simulink library "Renault_Library"  (Simulink 9.2)
settings: Solver=ode3  StartTime=0.0  StopTime=10.0  FixedStep=0.020  RelTol=1e-3  EnableMultiTasking=on
undefined symbols (not defined in the file or in its folder): ABC, AssystemProposal, b, chkval, CoeffA_C, CoeffB_C, CoeffMean_C, ConfTime_C, DiagDelay_C, DividePercent, FailureConfActive_C, FallingEdgeDuration_C, HighLevelThresholdVoltage_C, HighLevelVoltage_C, HighLevelVoltageTunable_C, InitValue_C, InputVoltRangeMax_C, InputVoltRangeMin_C, LowActiveContact_C, LowLevelThresholdVoltage_C, LowLevelVoltage_C, MaxOperatingVoltage_C, MinOperatingVoltage_C, mV2V_conversion, OUT1, OutOfRangeHigh_C, OutOfRangeLow_C, PinSupplySaturationMax, PinSupplySaturationMin, Res1Value_C, Res2Value_C, Res3Value_C, RisingEdgeDuration_C, RSA_CANSignalFailMonitoring, SampleTime_C, service14Table, service19Table, service22Table, service24Table, service28Table, service2ETable, service2FTable, service31Table, service87Table, SizeXStateDID2FTable, SizeXTable14, SizeXTable19, SizeXTable22, SizeXTable24, SizeXTable28, SizeXTable2E, SizeXTable2F, SizeXTable31, SizeXTable87, SizeYStateDID2FTable, SizeYTable14, SizeYTable19, SizeYTable22, SizeYTable24, SizeYTable28 ... (+22)
undefined symbols (not defined in the file): BatteryVoltage_ActivationThreshold_C, FrameStatus_AbsentValue_C, FrameStatus_ConfAbsentValue_C, MemThreshold_C, NAMEOFTHEMODEL_SAMPLE_TIME_CST, NbAbs1_C, NbConfAbs1_C, NbConfPres1_C, NbPres1_C, NOMDUSIGNAL_FaultConfTime_C, NOMDUSIGNAL_FaultRehabTime_C, NOMDUSIGNAL_switchvalue_C, NOMDUSIGNAL_unavailablevalue_C, PeriodFrame1_C, ResetTime_C, WakeUpTime_C
libraries: $bdroot x112, simulink x75
(part 26 of 32)

== system Renault_Library/Services ECU/Diag/RSA_DiagnosticManagement/DIAGNOSTIC_SANCTION_TOP/Service19/SubFunction_In_Active_Session_Service19/SubsystemDefaultSession_19 ==
  Active_Session : Inport
  Number_Subfunction : Inport  Port=2
  Constant{1..3,8,11,13,15,18..20,22,24,27..28,34,36,40..41,43,45,47,58,61} (23 alike) : Constant  Value=true  OutDataTypeStr=boolean  SampleTime=-1
  Constant10 : Constant  Value=ReporDTCSnapshotRecordByDTCNumberSession1  OutDataTypeStr=Inherit: Inherit via back propagation  SampleTime=-1
  Constant12 : Constant  Value=ReportNumberOfDTCByStatusMaskSession1  OutDataTypeStr=Inherit: Inherit via back propagation  SampleTime=-1
  Constant14 : Constant  Value=ReportDTCByStatusMaskSession1  OutDataTypeStr=Inherit: Inherit via back propagation  SampleTime=-1
  Constant16 : Constant  Value=ReporDTCSnapshotIdentificationSession1  OutDataTypeStr=Inherit: Inherit via back propagation  SampleTime=-1
  Constant17 : Constant  Value=ReportDTCStoredDataByRecordNumberSession1  OutDataTypeStr=Inherit: Inherit via back propagation  SampleTime=-1
  Constant21 : Constant  Value=ReportNumberOfDTCBySeverityMaskRecordSession1  OutDataTypeStr=Inherit: Inherit via back propagation  SampleTime=-1
  Constant23 : Constant  Value=ReportDTCBySeverityMaskRecordSession1  OutDataTypeStr=Inherit: Inherit via back propagation  SampleTime=-1
  Constant25 : Constant  Value=ReportSeverityInformationOfDTCSession1  OutDataTypeStr=Inherit: Inherit via back propagation  SampleTime=-1
  Constant26 : Constant  Value=ReportMostRecentConfimedDTCSession1  OutDataTypeStr=Inherit: Inherit via back propagation  SampleTime=-1
  Constant29 : Constant  Value=10  OutDataTypeStr=Inherit: Inherit via back propagation  SampleTime=-1
  Constant30 : Constant  Value=ReportFirstTestFailedDTCSession1  OutDataTypeStr=Inherit: Inherit via back propagation  SampleTime=-1
  Constant31 : Constant  Value=9  OutDataTypeStr=Inherit: Inherit via back propagation  SampleTime=-1
  Constant32 : Constant  Value=8  OutDataTypeStr=Inherit: Inherit via back propagation  SampleTime=-1
  Constant33 : Constant  Value=7  OutDataTypeStr=Inherit: Inherit via back propagation  SampleTime=-1
  Constant35 : Constant  Value=ReportFirstConfirmedDTCSession1  OutDataTypeStr=Inherit: Inherit via back propagation  SampleTime=-1
  Constant37 : Constant  Value=ReportMostRecentTestFailedDTCSession1  OutDataTypeStr=Inherit: Inherit via back propagation  SampleTime=-1
  Constant38 : Constant  Value=ReportMirrorMemoryDTCByStatusMaskSession1  OutDataTypeStr=Inherit: Inherit via back propagation  SampleTime=-1
  Constant4 : Constant  Value=4  OutDataTypeStr=Inherit: Inherit via back propagation  SampleTime=-1
  Constant42 : Constant  Value=ReportNumberOfMirrorMemoryDTCByStatusMaskSession1  OutDataTypeStr=Inherit: Inherit via back propagation  SampleTime=-1
  Constant44 : Constant  Value=ReportNumberOfEmissionsRelatedOBDDTCByStatusMaskSession1  OutDataTypeStr=Inherit: Inherit via back propagation  SampleTime=-1
  Constant46 : Constant  Value=ReportEmissionsRelatedOBDDTCByStatusMaskSession1  OutDataTypeStr=Inherit: Inherit via back propagation  SampleTime=-1
  Constant48 : Constant  Value=20  OutDataTypeStr=Inherit: Inherit via back propagation  SampleTime=-1
  Constant49 : Constant  Value=19  OutDataTypeStr=Inherit: Inherit via back propagation  SampleTime=-1
  Constant5 : Constant  Value=5  OutDataTypeStr=Inherit: Inherit via back propagation  SampleTime=-1
  Constant50 : Constant  Value=18  OutDataTypeStr=Inherit: Inherit via back propagation  SampleTime=-1
  Constant51 : Constant  Value=17  OutDataTypeStr=Inherit: Inherit via back propagation  SampleTime=-1
  Constant52 : Constant  Value=14  OutDataTypeStr=Inherit: Inherit via back propagation  SampleTime=-1
  Constant53 : Constant  Value=15  OutDataTypeStr=Inherit: Inherit via back propagation  SampleTime=-1
  Constant54 : Constant  Value=13  OutDataTypeStr=Inherit: Inherit via back propagation  SampleTime=-1
  Constant55 : Constant  Value=12  OutDataTypeStr=Inherit: Inherit via back propagation  SampleTime=-1
  Constant56 : Constant  Value=11  OutDataTypeStr=Inherit: Inherit via back propagation  SampleTime=-1
  Constant57 : Constant  Value=ReportMirrorMemoryDTCExtendedDataRecordByDTCNumberSession1  OutDataTypeStr=Inherit: Inherit via back propagation  SampleTime=-1
  Constant59 : Constant  Value=16  OutDataTypeStr=Inherit: Inherit via back propagation  SampleTime=-1
  Constant6 : Constant  Value=3  OutDataTypeStr=Inherit: Inherit via back propagation  SampleTime=-1
  Constant60 : Constant  Value=ReportDTCFaultDetectionCounterSession1  OutDataTypeStr=Inherit: Inherit via back propagation  SampleTime=-1
  Constant62 : Constant  Value=ReportDTCWithPermanetStatusSession1  OutDataTypeStr=Inherit: Inherit via back propagation  SampleTime=-1
  Constant64 : Constant  Value=ReportWWHOBDDTCByMaskRecordSession1  OutDataTypeStr=Inherit: Inherit via back propagation  SampleTime=-1
  Constant65 : Constant  Value=2  OutDataTypeStr=Inherit: Inherit via back propagation  SampleTime=-1
  Constant67 : Constant  Value=85  OutDataTypeStr=Inherit: Inherit via back propagation  SampleTime=-1
  Constant68 : Constant  Value=66  OutDataTypeStr=Inherit: Inherit via back propagation  SampleTime=-1
  Constant69 : Constant  Value=21  OutDataTypeStr=Inherit: Inherit via back propagation  SampleTime=-1
  Constant7 : Constant  OutDataTypeStr=Inherit: Inherit via back propagation  SampleTime=-1
  Constant70 : Constant  Value=ReportWWHOBDDTCWithPermanentStatusSession1  OutDataTypeStr=Inherit: Inherit via back propagation  SampleTime=-1
  Constant74 : Constant  Value=ReportDTCExtendedDataRecordByDTCNumberSession1  OutDataTypeStr=Inherit: Inherit via back propagation  SampleTime=-1
  Constant76 : Constant  Value=6  OutDataTypeStr=Inherit: Inherit via back propagation  SampleTime=-1
  Constant9 : Constant  Value=ReportSupportedDTCSession1  OutDataTypeStr=Inherit: Inherit via back propagation  SampleTime=-1
  Logical Operator{1..22,24} (23 alike) : Logic  OutDataTypeStr=boolean
  LogicalOperator{,1..3,5..22,28} (23 alike) : Logic  OutDataTypeStr=boolean
  LogicalOperator4 : Logic  Operator=OR  Inputs=23  OutDataTypeStr=boolean
  RelationalOperator{4..8,10..48,51..52} (46 alike) : RelationalOperator  Operator===  OutDataTypeStr=boolean  RndMeth=Nearest  InputSameDT=on
  Out1 : Outport
  wires:
  Constant7/1 -> RelationalOperator7/2
  Constant12/1 -> RelationalOperator10/1
  Constant1/1 -> RelationalOperator10/2
  RelationalOperator10/1 -> LogicalOperator/1
  Constant24/1 -> RelationalOperator11/2
  Constant14/1 -> RelationalOperator11/1
  RelationalOperator11/1 -> LogicalOperator1/1
  Constant40/1 -> RelationalOperator12/2
  Constant16/1 -> RelationalOperator12/1
  RelationalOperator12/1 -> LogicalOperator2/1
  Constant10/1 -> RelationalOperator14/1
  Constant41/1 -> RelationalOperator14/2
  RelationalOperator14/1 -> LogicalOperator3/1
  Constant17/1 -> RelationalOperator13/1
  Constant43/1 -> RelationalOperator13/2
  RelationalOperator13/1 -> LogicalOperator6/1
  Logical Operator2/1 -> LogicalOperator1/2
  Logical Operator1/1 -> LogicalOperator/2
  Active_Session/1 -> Logical Operator10/2, Logical Operator22/2, Logical Operator19/2, Logical Operator21/2, Logical Operator20/2, Logical Operator18/2, Logical Operator17/2, Logical Operator13/2, Logical Operator15/2, Logical Operator14/2, Logical Operator16/2, Logical Operator12/2, Logical Operator8/2, Logical Operator9/2, Logical Operator11/2, Logical Operator7/2, Logical Operator6/2, Logical Operator24/2, Logical Operator5/2, Logical Operator4/2, Logical Operator3/2, Logical Operator2/2, Logical Operator1/2
  Constant65/1 -> RelationalOperator45/2
  RelationalOperator45/1 -> Logical Operator2/1
  Constant4/1 -> RelationalOperator4/2
  Constant5/1 -> RelationalOperator5/2
  RelationalOperator5/1 -> Logical Operator4/1
  Logical Operator4/1 -> LogicalOperator6/2
  Logical Operator3/1 -> LogicalOperator3/2
  Constant6/1 -> RelationalOperator6/2
  Logical Operator5/1 -> LogicalOperator2/2
  RelationalOperator7/1 -> Logical Operator1/1
  RelationalOperator4/1 -> Logical Operator3/1
  RelationalOperator6/1 -> Logical Operator5/1
  [Number_Subfunction] -> RelationalOperator7/1
  [Number_Subfunction] -> RelationalOperator45/1
  [Number_Subfunction] -> RelationalOperator6/1
  [Number_Subfunction] -> RelationalOperator4/1
  [Number_Subfunction] -> RelationalOperator5/1
  Constant74/1 -> RelationalOperator51/1
  Constant45/1 -> RelationalOperator51/2
  RelationalOperator51/1 -> LogicalOperator28/1
  Constant76/1 -> RelationalOperator52/2
  RelationalOperator52/1 -> Logical Operator24/1
  Logical Operator24/1 -> LogicalOperator28/2
  [Number_Subfunction] -> RelationalOperator52/1
  Constant33/1 -> RelationalOperator25/2
  Constant21/1 -> RelationalOperator8/1
  Constant47/1 -> RelationalOperator8/2
  RelationalOperator8/1 -> LogicalOperator5/1
  Constant58/1 -> RelationalOperator15/2
  Constant23/1 -> RelationalOperator15/1
  RelationalOperator15/1 -> LogicalOperator7/1
  Constant61/1 -> RelationalOperator16/2
  Constant25/1 -> RelationalOperator16/1
  RelationalOperator16/1 -> LogicalOperator8/1
  Constant9/1 -> RelationalOperator18/1
  Constant2/1 -> RelationalOperator18/2
  RelationalOperator18/1 -> LogicalOperator10/1
  Logical Operator7/1 -> LogicalOperator7/2
  Logical Operator6/1 -> LogicalOperator5/2
  Constant32/1 -> RelationalOperator20/2
  RelationalOperator20/1 -> Logical Operator7/1
  Constant29/1 -> RelationalOperator19/2
  Logical Operator9/1 -> LogicalOperator10/2
  Constant31/1 -> RelationalOperator24/2
  Logical Operator11/1 -> LogicalOperator8/2
  RelationalOperator25/1 -> Logical Operator6/1
  RelationalOperator19/1 -> Logical Operator9/1
  RelationalOperator24/1 -> Logical Operator11/1
  [Number_Subfunction] -> RelationalOperator25/1
  [Number_Subfunction] -> RelationalOperator20/1
  [Number_Subfunction] -> RelationalOperator24/1
  [Number_Subfunction] -> RelationalOperator19/1
  Constant56/1 -> RelationalOperator40/2
  Constant30/1 -> RelationalOperator17/1
  Constant11/1 -> RelationalOperator17/2
  RelationalOperator17/1 -> LogicalOperator9/1
  Constant3/1 -> RelationalOperator21/2
  Constant35/1 -> RelationalOperator21/1
  RelationalOperator21/1 -> LogicalOperator11/1
  Constant13/1 -> RelationalOperator22/2
  Constant37/1 -> RelationalOperator22/1
  RelationalOperator22/1 -> LogicalOperator13/1
  Constant26/1 -> RelationalOperator26/1
  Constant8/1 -> RelationalOperator26/2
  RelationalOperator26/1 -> LogicalOperator15/1
  Constant38/1 -> RelationalOperator23/1
  Constant15/1 -> RelationalOperator23/2
  RelationalOperator23/1 -> LogicalOperator17/1
  Logical Operator12/1 -> LogicalOperator11/2
  Logical Operator8/1 -> LogicalOperator9/2
  Constant55/1 -> RelationalOperator35/2
  RelationalOperator35/1 -> Logical Operator12/1
  Constant52/1 -> RelationalOperator34/2
  Constant53/1 -> RelationalOperator36/2
  RelationalOperator36/1 -> Logical Operator15/1
  Logical Operator15/1 -> LogicalOperator17/2
  Logical Operator14/1 -> LogicalOperator15/2
  Constant54/1 -> RelationalOperator39/2
  Logical Operator16/1 -> LogicalOperator13/2
  RelationalOperator40/1 -> Logical Operator8/1
  RelationalOperator34/1 -> Logical Operator14/1
  RelationalOperator39/1 -> Logical Operator16/1
  [Number_Subfunction] -> RelationalOperator40/1
  [Number_Subfunction] -> RelationalOperator35/1
  [Number_Subfunction] -> RelationalOperator39/1
  [Number_Subfunction] -> RelationalOperator34/1
  [Number_Subfunction] -> RelationalOperator36/1
  Constant57/1 -> RelationalOperator37/1
  Constant18/1 -> RelationalOperator37/2
  RelationalOperator37/1 -> LogicalOperator14/1
  Constant59/1 -> RelationalOperator38/2
  RelationalOperator38/1 -> Logical Operator13/1
  Logical Operator13/1 -> LogicalOperator14/2
  [Number_Subfunction] -> RelationalOperator38/1
  Constant51/1 -> RelationalOperator33/2
  Constant42/1 -> RelationalOperator41/1
  Constant19/1 -> RelationalOperator41/2
  RelationalOperator41/1 -> LogicalOperator16/1
  Constant20/1 -> RelationalOperator27/2
  Constant44/1 -> RelationalOperator27/1
  RelationalOperator27/1 -> LogicalOperator18/1
  Constant22/1 -> RelationalOperator28/2
  Constant46/1 -> RelationalOperator28/1
  RelationalOperator28/1 -> LogicalOperator19/1
  Constant60/1 -> RelationalOperator29/1
  Constant27/1 -> RelationalOperator29/2
  RelationalOperator29/1 -> LogicalOperator12/1
  Logical Operator18/1 -> LogicalOperator18/2
  Logical Operator17/1 -> LogicalOperator16/2
  Constant50/1 -> RelationalOperator31/2
  RelationalOperator31/1 -> Logical Operator18/1
  Constant48/1 -> RelationalOperator30/2
  Logical Operator19/1 -> LogicalOperator12/2
  Constant49/1 -> RelationalOperator32/2
  Logical Operator10/1 -> LogicalOperator19/2
  RelationalOperator33/1 -> Logical Operator17/1
  RelationalOperator30/1 -> Logical Operator19/1
  RelationalOperator32/1 -> Logical Operator10/1
  [Number_Subfunction] -> RelationalOperator33/1
  [Number_Subfunction] -> RelationalOperator31/1
  [Number_Subfunction] -> RelationalOperator32/1
  [Number_Subfunction] -> RelationalOperator30/1
  Constant28/1 -> RelationalOperator42/2
  Constant62/1 -> RelationalOperator42/1
  RelationalOperator42/1 -> LogicalOperator21/1
  Constant34/1 -> RelationalOperator43/2
  Constant64/1 -> RelationalOperator43/1
  RelationalOperator43/1 -> LogicalOperator22/1
  Constant70/1 -> RelationalOperator44/1
  Constant36/1 -> RelationalOperator44/2
  RelationalOperator44/1 -> LogicalOperator20/1
  Logical Operator21/1 -> LogicalOperator21/2
  Constant69/1 -> RelationalOperator47/2
  RelationalOperator47/1 -> Logical Operator21/1
  Constant67/1 -> RelationalOperator46/2
  Logical Operator22/1 -> LogicalOperator20/2
  Constant68/1 -> RelationalOperator48/2
  Logical Operator20/1 -> LogicalOperator22/2
  RelationalOperator46/1 -> Logical Operator22/1
  RelationalOperator48/1 -> Logical Operator20/1
  [Number_Subfunction] -> RelationalOperator47/1
  [Number_Subfunction] -> RelationalOperator48/1
  [Number_Subfunction] -> RelationalOperator46/1
  LogicalOperator/1 -> LogicalOperator4/1
  LogicalOperator1/1 -> LogicalOperator4/2
  LogicalOperator2/1 -> LogicalOperator4/3
  LogicalOperator3/1 -> LogicalOperator4/4
  LogicalOperator6/1 -> LogicalOperator4/5
  LogicalOperator28/1 -> LogicalOperator4/6
  LogicalOperator5/1 -> LogicalOperator4/7
  LogicalOperator7/1 -> LogicalOperator4/8
  LogicalOperator8/1 -> LogicalOperator4/9
  LogicalOperator10/1 -> LogicalOperator4/10
  LogicalOperator9/1 -> LogicalOperator4/11
  LogicalOperator11/1 -> LogicalOperator4/12
  LogicalOperator13/1 -> LogicalOperator4/13
  LogicalOperator15/1 -> LogicalOperator4/14
  LogicalOperator17/1 -> LogicalOperator4/15
  LogicalOperator14/1 -> LogicalOperator4/16
  LogicalOperator16/1 -> LogicalOperator4/17
  LogicalOperator18/1 -> LogicalOperator4/18
  LogicalOperator19/1 -> LogicalOperator4/19
  LogicalOperator12/1 -> LogicalOperator4/20
  LogicalOperator21/1 -> LogicalOperator4/21
  LogicalOperator22/1 -> LogicalOperator4/22
  LogicalOperator20/1 -> LogicalOperator4/23
  LogicalOperator4/1 -> Out1/1
  Number_Subfunction/1 -> [Number_Subfunction]

== system Renault_Library/Services ECU/Diag/RSA_DiagnosticManagement/DIAGNOSTIC_SANCTION_TOP/Service19/SubFunction_In_Active_Session_Service19/SubsystemExtenderdSession_19 ==
  Active_Session : Inport
  Number_Subfunction : Inport  Port=2
  Constant{1..3,8,11,13,15,18..20,22,24,27..28,34,36,40..41,43,45,47,58,61} (23 alike) : Constant  Value=true  OutDataTypeStr=boolean  SampleTime=-1
  Constant144 : Constant  Value=ReportMostRecentTestFailedDTCSession3  OutDataTypeStr=Inherit: Inherit via back propagation  SampleTime=-1
  Constant145 : Constant  Value=ReportMirrorMemoryDTCByStatusMaskSession3  OutDataTypeStr=Inherit: Inherit via back propagation  SampleTime=-1
  Constant147 : Constant  Value=4  OutDataTypeStr=Inherit: Inherit via back propagation  SampleTime=-1
  Constant150 : Constant  Value=ReportNumberOfMirrorMemoryDTCByStatusMaskSession3  OutDataTypeStr=Inherit: Inherit via back propagation  SampleTime=-1
  Constant152 : Constant  Value=ReportNumberOfEmissionsRelatedOBDDTCByStatusMaskSession3  OutDataTypeStr=Inherit: Inherit via back propagation  SampleTime=-1
  Constant154 : Constant  Value=ReportEmissionsRelatedOBDDTCByStatusMaskSession3  OutDataTypeStr=Inherit: Inherit via back propagation  SampleTime=-1
  Constant156 : Constant  Value=20  OutDataTypeStr=Inherit: Inherit via back propagation  SampleTime=-1
  Constant157 : Constant  Value=19  OutDataTypeStr=Inherit: Inherit via back propagation  SampleTime=-1
  Constant158 : Constant  Value=5  OutDataTypeStr=Inherit: Inherit via back propagation  SampleTime=-1
  Constant159 : Constant  Value=18  OutDataTypeStr=Inherit: Inherit via back propagation  SampleTime=-1
  Constant160 : Constant  Value=17  OutDataTypeStr=Inherit: Inherit via back propagation  SampleTime=-1
  Constant161 : Constant  Value=14  OutDataTypeStr=Inherit: Inherit via back propagation  SampleTime=-1
  Constant162 : Constant  Value=15  OutDataTypeStr=Inherit: Inherit via back propagation  SampleTime=-1
  Constant163 : Constant  Value=13  OutDataTypeStr=Inherit: Inherit via back propagation  SampleTime=-1
  Constant164 : Constant  Value=12  OutDataTypeStr=Inherit: Inherit via back propagation  SampleTime=-1
  Constant165 : Constant  Value=11  OutDataTypeStr=Inherit: Inherit via back propagation  SampleTime=-1
  Constant166 : Constant  Value=ReportMirrorMemoryDTCExtendedDataRecordByDTCNumberSession3  OutDataTypeStr=Inherit: Inherit via back propagation  SampleTime=-1
  Constant168 : Constant  Value=16  OutDataTypeStr=Inherit: Inherit via back propagation  SampleTime=-1
  Constant169 : Constant  Value=3  OutDataTypeStr=Inherit: Inherit via back propagation  SampleTime=-1
  Constant170 : Constant  Value=ReportDTCFaultDetectionCounterSession3  OutDataTypeStr=Inherit: Inherit via back propagation  SampleTime=-1
  Constant172 : Constant  Value=ReportDTCWithPermanetStatusSession3  OutDataTypeStr=Inherit: Inherit via back propagation  SampleTime=-1
  Constant174 : Constant  Value=ReportWWHOBDDTCByMaskRecordSession3  OutDataTypeStr=Inherit: Inherit via back propagation  SampleTime=-1
  Constant175 : Constant  Value=2  OutDataTypeStr=Inherit: Inherit via back propagation  SampleTime=-1
  Constant177 : Constant  Value=85  OutDataTypeStr=Inherit: Inherit via back propagation  SampleTime=-1
  Constant178 : Constant  Value=66  OutDataTypeStr=Inherit: Inherit via back propagation  SampleTime=-1
  Constant179 : Constant  Value=21  OutDataTypeStr=Inherit: Inherit via back propagation  SampleTime=-1
  Constant180 : Constant  OutDataTypeStr=Inherit: Inherit via back propagation  SampleTime=-1
  Constant181 : Constant  Value=ReportWWHOBDDTCWithPermanentStatusSession3  OutDataTypeStr=Inherit: Inherit via back propagation  SampleTime=-1
  Constant182 : Constant  Value=ReportDTCExtendedDataRecordByDTCNumberSession3  OutDataTypeStr=Inherit: Inherit via back propagation  SampleTime=-1
  Constant184 : Constant  Value=6  OutDataTypeStr=Inherit: Inherit via back propagation  SampleTime=-1
  Constant185 : Constant  Value=ReportSupportedDTCSession3  OutDataTypeStr=Inherit: Inherit via back propagation  SampleTime=-1
  Constant186 : Constant  Value=ReporDTCSnapshotRecordByDTCNumberSession3  OutDataTypeStr=Inherit: Inherit via back propagation  SampleTime=-1
  Constant188 : Constant  Value=ReportNumberOfDTCByStatusMaskSession3  OutDataTypeStr=Inherit: Inherit via back propagation  SampleTime=-1
  Constant190 : Constant  Value=ReportDTCByStatusMaskSession3  OutDataTypeStr=Inherit: Inherit via back propagation  SampleTime=-1
  Constant192 : Constant  Value=ReporDTCSnapshotIdentificationSession3  OutDataTypeStr=Inherit: Inherit via back propagation  SampleTime=-1
  Constant193 : Constant  Value=ReportDTCStoredDataByRecordNumberSession3  OutDataTypeStr=Inherit: Inherit via back propagation  SampleTime=-1
  Constant197 : Constant  Value=ReportNumberOfDTCBySeverityMaskRecordSession3  OutDataTypeStr=Inherit: Inherit via back propagation  SampleTime=-1
  Constant199 : Constant  Value=ReportDTCBySeverityMaskRecordSession3  OutDataTypeStr=Inherit: Inherit via back propagation  SampleTime=-1
  Constant201 : Constant  Value=ReportSeverityInformationOfDTCSession3  OutDataTypeStr=Inherit: Inherit via back propagation  SampleTime=-1
  Constant202 : Constant  Value=ReportMostRecentConfimedDTCSession3  OutDataTypeStr=Inherit: Inherit via back propagation  SampleTime=-1
  Constant205 : Constant  Value=10  OutDataTypeStr=Inherit: Inherit via back propagation  SampleTime=-1
  Constant206 : Constant  Value=ReportFirstTestFailedDTCSession3  OutDataTypeStr=Inherit: Inherit via back propagation  SampleTime=-1
  Constant207 : Constant  Value=9  OutDataTypeStr=Inherit: Inherit via back propagation  SampleTime=-1
  Constant208 : Constant  Value=8  OutDataTypeStr=Inherit: Inherit via back propagation  SampleTime=-1
  Constant209 : Constant  Value=7  OutDataTypeStr=Inherit: Inherit via back propagation  SampleTime=-1
  Constant211 : Constant  Value=ReportFirstConfirmedDTCSession3  OutDataTypeStr=Inherit: Inherit via back propagation  SampleTime=-1
  Logical Operator{47..69} (23 alike) : Logic  OutDataTypeStr=boolean
  LogicalOperator{49..66,68..72} (23 alike) : Logic  OutDataTypeStr=boolean
  LogicalOperator67 : Logic  Operator=OR  Inputs=23  OutDataTypeStr=boolean
  RelationalOperator{97..142} (46 alike) : RelationalOperator  Operator===  OutDataTypeStr=boolean  RndMeth=Nearest  InputSameDT=on
  Out1 : Outport
  wires:
  Constant180/1 -> RelationalOperator141/2
  Constant188/1 -> RelationalOperator97/1
  Constant1/1 -> RelationalOperator97/2
  RelationalOperator97/1 -> LogicalOperator49/1
  Constant24/1 -> RelationalOperator98/2
  Constant190/1 -> RelationalOperator98/1
  RelationalOperator98/1 -> LogicalOperator50/1
  Constant40/1 -> RelationalOperator99/2
  Constant192/1 -> RelationalOperator99/1
  RelationalOperator99/1 -> LogicalOperator61/1
  Constant186/1 -> RelationalOperator101/1
  Constant41/1 -> RelationalOperator101/2
  RelationalOperator101/1 -> LogicalOperator66/1
  Constant193/1 -> RelationalOperator100/1
  Constant43/1 -> RelationalOperator100/2
  RelationalOperator100/1 -> LogicalOperator69/1
  Logical Operator58/1 -> LogicalOperator50/2
  Logical Operator47/1 -> LogicalOperator49/2
  Active_Session/1 -> Logical Operator48/2, Logical Operator61/2, Logical Operator57/2, Logical Operator60/2, Logical Operator59/2, Logical Operator56/2, Logical Operator55/2, Logical Operator51/2, Logical Operator53/2, Logical Operator52/2, Logical Operator54/2, Logical Operator50/2, Logical Operator68/2, Logical Operator69/2, Logical Operator49/2, Logical Operator67/2, Logical Operator66/2, Logical Operator62/2, Logical Operator65/2, Logical Operator64/2, Logical Operator63/2, Logical Operator58/2, Logical Operator47/2
  Constant175/1 -> RelationalOperator133/2
  RelationalOperator133/1 -> Logical Operator58/1
  Constant147/1 -> RelationalOperator127/2
  Constant158/1 -> RelationalOperator137/2
  RelationalOperator137/1 -> Logical Operator64/1
  Logical Operator64/1 -> LogicalOperator69/2
  Logical Operator63/1 -> LogicalOperator66/2
  Constant169/1 -> RelationalOperator140/2
  Logical Operator65/1 -> LogicalOperator61/2
  RelationalOperator141/1 -> Logical Operator47/1
  RelationalOperator127/1 -> Logical Operator63/1
  RelationalOperator140/1 -> Logical Operator65/1
  [Number_Subfunction] -> RelationalOperator141/1
  [Number_Subfunction] -> RelationalOperator133/1
  [Number_Subfunction] -> RelationalOperator140/1
  [Number_Subfunction] -> RelationalOperator127/1
  [Number_Subfunction] -> RelationalOperator137/1
  Constant182/1 -> RelationalOperator138/1
  ? -> RelationalOperator138/2
  RelationalOperator138/1 -> LogicalOperator65/1
  Constant184/1 -> RelationalOperator139/2
  RelationalOperator139/1 -> Logical Operator62/1
  Logical Operator62/1 -> LogicalOperator65/2
  [Number_Subfunction] -> RelationalOperator139/1
  Constant209/1 -> RelationalOperator112/2
  Constant197/1 -> RelationalOperator142/1
  Constant47/1 -> RelationalOperator142/2
  RelationalOperator142/1 -> LogicalOperator68/1
  Constant58/1 -> RelationalOperator102/2
  Constant199/1 -> RelationalOperator102/1
  RelationalOperator102/1 -> LogicalOperator70/1
  Constant61/1 -> RelationalOperator103/2
  Constant201/1 -> RelationalOperator103/1
  RelationalOperator103/1 -> LogicalOperator71/1
  Constant185/1 -> RelationalOperator105/1
  Constant2/1 -> RelationalOperator105/2
  RelationalOperator105/1 -> LogicalOperator51/1
  Logical Operator67/1 -> LogicalOperator70/2
  Logical Operator66/1 -> LogicalOperator68/2
  Constant208/1 -> RelationalOperator107/2
  RelationalOperator107/1 -> Logical Operator67/1
  Constant205/1 -> RelationalOperator106/2
  Logical Operator69/1 -> LogicalOperator51/2
  Constant207/1 -> RelationalOperator111/2
  Logical Operator49/1 -> LogicalOperator71/2
  RelationalOperator112/1 -> Logical Operator66/1
  RelationalOperator106/1 -> Logical Operator69/1
  RelationalOperator111/1 -> Logical Operator49/1
  [Number_Subfunction] -> RelationalOperator112/1
  [Number_Subfunction] -> RelationalOperator107/1
  [Number_Subfunction] -> RelationalOperator111/1
  [Number_Subfunction] -> RelationalOperator106/1
  Constant165/1 -> RelationalOperator128/2
  Constant206/1 -> RelationalOperator104/1
  Constant11/1 -> RelationalOperator104/2
  RelationalOperator104/1 -> LogicalOperator72/1
  Constant3/1 -> RelationalOperator108/2
  Constant211/1 -> RelationalOperator108/1
  RelationalOperator108/1 -> LogicalOperator52/1
  Constant13/1 -> RelationalOperator109/2
  Constant144/1 -> RelationalOperator109/1
  RelationalOperator109/1 -> LogicalOperator54/1
  Constant202/1 -> RelationalOperator113/1
  Constant8/1 -> RelationalOperator113/2
  RelationalOperator113/1 -> LogicalOperator56/1
  Constant145/1 -> RelationalOperator110/1
  Constant15/1 -> RelationalOperator110/2
  RelationalOperator110/1 -> LogicalOperator58/1
  Logical Operator50/1 -> LogicalOperator52/2
  Logical Operator68/1 -> LogicalOperator72/2
  Constant164/1 -> RelationalOperator122/2
  RelationalOperator122/1 -> Logical Operator50/1
  Constant161/1 -> RelationalOperator121/2
  Constant162/1 -> RelationalOperator123/2
  RelationalOperator123/1 -> Logical Operator53/1
  Logical Operator53/1 -> LogicalOperator58/2
  Logical Operator52/1 -> LogicalOperator56/2
  Constant163/1 -> RelationalOperator126/2
  Logical Operator54/1 -> LogicalOperator54/2
  RelationalOperator128/1 -> Logical Operator68/1
  RelationalOperator121/1 -> Logical Operator52/1
  RelationalOperator126/1 -> Logical Operator54/1
  [Number_Subfunction] -> RelationalOperator128/1
  [Number_Subfunction] -> RelationalOperator122/1
  [Number_Subfunction] -> RelationalOperator126/1
  [Number_Subfunction] -> RelationalOperator121/1
  [Number_Subfunction] -> RelationalOperator123/1
  Constant166/1 -> RelationalOperator124/1
  Constant18/1 -> RelationalOperator124/2
  RelationalOperator124/1 -> LogicalOperator55/1
  Constant168/1 -> RelationalOperator125/2
  RelationalOperator125/1 -> Logical Operator51/1
  Logical Operator51/1 -> LogicalOperator55/2
  [Number_Subfunction] -> RelationalOperator125/1
  Constant160/1 -> RelationalOperator120/2
  Constant150/1 -> RelationalOperator129/1
  Constant19/1 -> RelationalOperator129/2
  RelationalOperator129/1 -> LogicalOperator57/1
  Constant20/1 -> RelationalOperator114/2
  Constant152/1 -> RelationalOperator114/1
  RelationalOperator114/1 -> LogicalOperator59/1
  Constant22/1 -> RelationalOperator115/2
  Constant154/1 -> RelationalOperator115/1
  RelationalOperator115/1 -> LogicalOperator60/1
  Constant170/1 -> RelationalOperator116/1
  Constant27/1 -> RelationalOperator116/2
  RelationalOperator116/1 -> LogicalOperator53/1
  Logical Operator56/1 -> LogicalOperator59/2
  Logical Operator55/1 -> LogicalOperator57/2
  Constant159/1 -> RelationalOperator118/2
  RelationalOperator118/1 -> Logical Operator56/1
  Constant156/1 -> RelationalOperator117/2
  Logical Operator57/1 -> LogicalOperator53/2
  Constant157/1 -> RelationalOperator119/2
  Logical Operator48/1 -> LogicalOperator60/2
  RelationalOperator120/1 -> Logical Operator55/1
  RelationalOperator117/1 -> Logical Operator57/1
  RelationalOperator119/1 -> Logical Operator48/1
  [Number_Subfunction] -> RelationalOperator120/1
  [Number_Subfunction] -> RelationalOperator118/1
  [Number_Subfunction] -> RelationalOperator119/1
  [Number_Subfunction] -> RelationalOperator117/1
  Constant28/1 -> RelationalOperator130/2
  Constant172/1 -> RelationalOperator130/1
  RelationalOperator130/1 -> LogicalOperator63/1
  Constant34/1 -> RelationalOperator131/2
  Constant174/1 -> RelationalOperator131/1
  RelationalOperator131/1 -> LogicalOperator64/1
  Constant181/1 -> RelationalOperator132/1
  Constant36/1 -> RelationalOperator132/2
  RelationalOperator132/1 -> LogicalOperator62/1
  Logical Operator60/1 -> LogicalOperator63/2
  Constant179/1 -> RelationalOperator135/2
  RelationalOperator135/1 -> Logical Operator60/1
  Constant177/1 -> RelationalOperator134/2
  Logical Operator61/1 -> LogicalOperator62/2
  Constant178/1 -> RelationalOperator136/2
  Logical Operator59/1 -> LogicalOperator64/2
  RelationalOperator134/1 -> Logical Operator61/1
  RelationalOperator136/1 -> Logical Operator59/1
  [Number_Subfunction] -> RelationalOperator135/1
  [Number_Subfunction] -> RelationalOperator136/1
  [Number_Subfunction] -> RelationalOperator134/1
  LogicalOperator49/1 -> LogicalOperator67/1
  LogicalOperator50/1 -> LogicalOperator67/2
  LogicalOperator61/1 -> LogicalOperator67/3
  LogicalOperator66/1 -> LogicalOperator67/4
  LogicalOperator69/1 -> LogicalOperator67/5
  LogicalOperator65/1 -> LogicalOperator67/6
  LogicalOperator68/1 -> LogicalOperator67/7
  LogicalOperator70/1 -> LogicalOperator67/8
  LogicalOperator71/1 -> LogicalOperator67/9
  LogicalOperator51/1 -> LogicalOperator67/10
  LogicalOperator72/1 -> LogicalOperator67/11
  LogicalOperator52/1 -> LogicalOperator67/12
  LogicalOperator54/1 -> LogicalOperator67/13
  LogicalOperator56/1 -> LogicalOperator67/14
  LogicalOperator58/1 -> LogicalOperator67/15
  LogicalOperator55/1 -> LogicalOperator67/16
  LogicalOperator57/1 -> LogicalOperator67/17
  LogicalOperator59/1 -> LogicalOperator67/18
  LogicalOperator60/1 -> LogicalOperator67/19
  LogicalOperator53/1 -> LogicalOperator67/20
  LogicalOperator63/1 -> LogicalOperator67/21
  LogicalOperator64/1 -> LogicalOperator67/22
  LogicalOperator62/1 -> LogicalOperator67/23
  LogicalOperator67/1 -> Out1/1
  Number_Subfunction/1 -> [Number_Subfunction]

== system Renault_Library/Services ECU/Diag/RSA_DiagnosticManagement/DIAGNOSTIC_SANCTION_TOP/Service19/SubFunction_In_Active_Session_Service19/SubsystemProgrammingSession_19 ==
  Active_Session : Inport
  Number_Subfunction : Inport  Port=2
  Constant{1..3,8,11,13,15,18..20,22,24,27..28,34,36,40..41,43,45,47,58,61} (23 alike) : Constant  Value=true  OutDataTypeStr=boolean  SampleTime=-1
  Constant101 : Constant  Value=ReportMostRecentTestFailedDTCSession2  OutDataTypeStr=Inherit: Inherit via back propagation  SampleTime=-1
  Constant102 : Constant  Value=ReportMirrorMemoryDTCByStatusMaskSession2  OutDataTypeStr=Inherit: Inherit via back propagation  SampleTime=-1
  Constant104 : Constant  Value=4  OutDataTypeStr=Inherit: Inherit via back propagation  SampleTime=-1
  Constant107 : Constant  Value=ReportNumberOfMirrorMemoryDTCByStatusMaskSession2  OutDataTypeStr=Inherit: Inherit via back propagation  SampleTime=-1
  Constant109 : Constant  Value=ReportNumberOfEmissionsRelatedOBDDTCByStatusMaskSession2  OutDataTypeStr=Inherit: Inherit via back propagation  SampleTime=-1
  Constant111 : Constant  Value=ReportEmissionsRelatedOBDDTCByStatusMaskSession2  OutDataTypeStr=Inherit: Inherit via back propagation  SampleTime=-1
  Constant113 : Constant  Value=20  OutDataTypeStr=Inherit: Inherit via back propagation  SampleTime=-1
  Constant114 : Constant  Value=19  OutDataTypeStr=Inherit: Inherit via back propagation  SampleTime=-1
  Constant115 : Constant  Value=5  OutDataTypeStr=Inherit: Inherit via back propagation  SampleTime=-1
  Constant116 : Constant  Value=18  OutDataTypeStr=Inherit: Inherit via back propagation  SampleTime=-1
  Constant117 : Constant  Value=17  OutDataTypeStr=Inherit: Inherit via back propagation  SampleTime=-1
  Constant118 : Constant  Value=14  OutDataTypeStr=Inherit: Inherit via back propagation  SampleTime=-1
  Constant119 : Constant  Value=15  OutDataTypeStr=Inherit: Inherit via back propagation  SampleTime=-1
  Constant120 : Constant  Value=13  OutDataTypeStr=Inherit: Inherit via back propagation  SampleTime=-1
  Constant121 : Constant  Value=12  OutDataTypeStr=Inherit: Inherit via back propagation  SampleTime=-1
  Constant122 : Constant  Value=11  OutDataTypeStr=Inherit: Inherit via back propagation  SampleTime=-1
  Constant123 : Constant  Value=ReportMirrorMemoryDTCExtendedDataRecordByDTCNumberSession2  OutDataTypeStr=Inherit: Inherit via back propagation  SampleTime=-1
  Constant125 : Constant  Value=16  OutDataTypeStr=Inherit: Inherit via back propagation  SampleTime=-1
  Constant126 : Constant  Value=3  OutDataTypeStr=Inherit: Inherit via back propagation  SampleTime=-1
  Constant127 : Constant  Value=ReportDTCFaultDetectionCounterSession2  OutDataTypeStr=Inherit: Inherit via back propagation  SampleTime=-1
  Constant129 : Constant  Value=ReportDTCWithPermanetStatusSession2  OutDataTypeStr=Inherit: Inherit via back propagation  SampleTime=-1
  Constant131 : Constant  Value=ReportWWHOBDDTCByMaskRecordSession2  OutDataTypeStr=Inherit: Inherit via back propagation  SampleTime=-1
  Constant132 : Constant  Value=2  OutDataTypeStr=Inherit: Inherit via back propagation  SampleTime=-1
  Constant134 : Constant  Value=85  OutDataTypeStr=Inherit: Inherit via back propagation  SampleTime=-1
  Constant135 : Constant  Value=66  OutDataTypeStr=Inherit: Inherit via back propagation  SampleTime=-1
  Constant136 : Constant  Value=21  OutDataTypeStr=Inherit: Inherit via back propagation  SampleTime=-1
  Constant137 : Constant  OutDataTypeStr=Inherit: Inherit via back propagation  SampleTime=-1
  Constant138 : Constant  Value=ReportWWHOBDDTCWithPermanentStatusSession2  OutDataTypeStr=Inherit: Inherit via back propagation  SampleTime=-1
  Constant139 : Constant  Value=ReportDTCExtendedDataRecordByDTCNumberSession2  OutDataTypeStr=Inherit: Inherit via back propagation  SampleTime=-1
  Constant141 : Constant  Value=6  OutDataTypeStr=Inherit: Inherit via back propagation  SampleTime=-1
  Constant142 : Constant  Value=ReportSupportedDTCSession2  OutDataTypeStr=Inherit: Inherit via back propagation  SampleTime=-1
  Constant71 : Constant  Value=ReporDTCSnapshotRecordByDTCNumberSession2  OutDataTypeStr=Inherit: Inherit via back propagation  SampleTime=-1
  Constant73 : Constant  Value=ReportNumberOfDTCByStatusMaskSession2  OutDataTypeStr=Inherit: Inherit via back propagation  SampleTime=-1
  Constant78 : Constant  Value=ReportDTCByStatusMaskSession2  OutDataTypeStr=Inherit: Inherit via back propagation  SampleTime=-1
  Constant80 : Constant  Value=ReporDTCSnapshotIdentificationSession2  OutDataTypeStr=Inherit: Inherit via back propagation  SampleTime=-1
  Constant81 : Constant  Value=ReportDTCStoredDataByRecordNumberSession2  OutDataTypeStr=Inherit: Inherit via back propagation  SampleTime=-1
  Constant85 : Constant  Value=ReportNumberOfDTCBySeverityMaskRecordSession2  OutDataTypeStr=Inherit: Inherit via back propagation  SampleTime=-1
  Constant87 : Constant  Value=ReportDTCBySeverityMaskRecordSession2  OutDataTypeStr=Inherit: Inherit via back propagation  SampleTime=-1
  Constant89 : Constant  Value=ReportSeverityInformationOfDTCSession2  OutDataTypeStr=Inherit: Inherit via back propagation  SampleTime=-1
  Constant90 : Constant  Value=ReportMostRecentConfimedDTCSession2  OutDataTypeStr=Inherit: Inherit via back propagation  SampleTime=-1
  Constant93 : Constant  Value=10  OutDataTypeStr=Inherit: Inherit via back propagation  SampleTime=-1
  Constant94 : Constant  Value=ReportFirstTestFailedDTCSession2  OutDataTypeStr=Inherit: Inherit via back propagation  SampleTime=-1
  Constant95 : Constant  Value=9  OutDataTypeStr=Inherit: Inherit via back propagation  SampleTime=-1
  Constant96 : Constant  Value=8  OutDataTypeStr=Inherit: Inherit via back propagation  SampleTime=-1
  Constant97 : Constant  Value=7  OutDataTypeStr=Inherit: Inherit via back propagation  SampleTime=-1
  Constant99 : Constant  Value=ReportFirstConfirmedDTCSession2  OutDataTypeStr=Inherit: Inherit via back propagation  SampleTime=-1
  Logical Operator{23,25..46} (23 alike) : Logic  OutDataTypeStr=boolean
  LogicalOperator{23,25..27,29..42,44..48} (23 alike) : Logic  OutDataTypeStr=boolean
  LogicalOperator43 : Logic  Operator=OR  Inputs=23  OutDataTypeStr=boolean
  RelationalOperator{49..50,53..96} (46 alike) : RelationalOperator  Operator===  OutDataTypeStr=boolean  RndMeth=Nearest  InputSameDT=on
  Out1 : Outport
  wires:
  Constant137/1 -> RelationalOperator95/2
  Constant73/1 -> RelationalOperator49/1
  Constant1/1 -> RelationalOperator49/2
  RelationalOperator49/1 -> LogicalOperator23/1
  Constant24/1 -> RelationalOperator50/2
  Constant78/1 -> RelationalOperator50/1
  RelationalOperator50/1 -> LogicalOperator25/1
  Constant40/1 -> RelationalOperator53/2
  Constant80/1 -> RelationalOperator53/1
  RelationalOperator53/1 -> LogicalOperator37/1
  Constant71/1 -> RelationalOperator55/1
  Constant41/1 -> RelationalOperator55/2
  RelationalOperator55/1 -> LogicalOperator42/1
  Constant81/1 -> RelationalOperator54/1
  Constant43/1 -> RelationalOperator54/2
  RelationalOperator54/1 -> LogicalOperator45/1
  Logical Operator35/1 -> LogicalOperator25/2
  Logical Operator23/1 -> LogicalOperator23/2
  Active_Session/1 -> Logical Operator23/2, Logical Operator35/2, Logical Operator40/2, Logical Operator41/2, Logical Operator42/2, Logical Operator39/2, Logical Operator43/2, Logical Operator44/2, Logical Operator26/2, Logical Operator46/2, Logical Operator45/2, Logical Operator27/2, Logical Operator31/2, Logical Operator29/2, Logical Operator30/2, Logical Operator28/2, Logical Operator32/2, Logical Operator33/2, Logical Operator36/2, Logical Operator37/2, Logical Operator34/2, Logical Operator38/2, Logical Operator25/2
  Constant132/1 -> RelationalOperator87/2
  RelationalOperator87/1 -> Logical Operator35/1
  Constant104/1 -> RelationalOperator81/2
  Constant115/1 -> RelationalOperator91/2
  RelationalOperator91/1 -> Logical Operator41/1
  Logical Operator41/1 -> LogicalOperator45/2
  Logical Operator40/1 -> LogicalOperator42/2
  Constant126/1 -> RelationalOperator94/2
  Logical Operator42/1 -> LogicalOperator37/2
  RelationalOperator95/1 -> Logical Operator23/1
  RelationalOperator81/1 -> Logical Operator40/1
  RelationalOperator94/1 -> Logical Operator42/1
  [Number_Subfunction] -> RelationalOperator95/1
  [Number_Subfunction] -> RelationalOperator87/1
  [Number_Subfunction] -> RelationalOperator94/1
  [Number_Subfunction] -> RelationalOperator81/1
  [Number_Subfunction] -> RelationalOperator91/1
  Constant139/1 -> RelationalOperator92/1
  Constant45/1 -> RelationalOperator92/2
  RelationalOperator92/1 -> LogicalOperator41/1
  Constant141/1 -> RelationalOperator93/2
  RelationalOperator93/1 -> Logical Operator39/1
  Logical Operator39/1 -> LogicalOperator41/2
  [Number_Subfunction] -> RelationalOperator93/1
  Constant97/1 -> RelationalOperator66/2
  Constant85/1 -> RelationalOperator96/1
  Constant47/1 -> RelationalOperator96/2
  RelationalOperator96/1 -> LogicalOperator44/1
  Constant58/1 -> RelationalOperator56/2
  Constant87/1 -> RelationalOperator56/1
  RelationalOperator56/1 -> LogicalOperator46/1
  Constant61/1 -> RelationalOperator57/2
  Constant89/1 -> RelationalOperator57/1
  RelationalOperator57/1 -> LogicalOperator47/1
  Constant142/1 -> RelationalOperator59/1
  Constant2/1 -> RelationalOperator59/2
  RelationalOperator59/1 -> LogicalOperator26/1
  Logical Operator44/1 -> LogicalOperator46/2
  Logical Operator43/1 -> LogicalOperator44/2
  Constant96/1 -> RelationalOperator61/2
  RelationalOperator61/1 -> Logical Operator44/1
  Constant93/1 -> RelationalOperator60/2
  Logical Operator46/1 -> LogicalOperator26/2
  Constant95/1 -> RelationalOperator65/2
  Logical Operator26/1 -> LogicalOperator47/2
  RelationalOperator66/1 -> Logical Operator43/1
  RelationalOperator60/1 -> Logical Operator46/1
  RelationalOperator65/1 -> Logical Operator26/1
  [Number_Subfunction] -> RelationalOperator66/1
  [Number_Subfunction] -> RelationalOperator61/1
  [Number_Subfunction] -> RelationalOperator65/1
  [Number_Subfunction] -> RelationalOperator60/1
  Constant122/1 -> RelationalOperator82/2
  Constant94/1 -> RelationalOperator58/1
  Constant11/1 -> RelationalOperator58/2
  RelationalOperator58/1 -> LogicalOperator48/1
  Constant3/1 -> RelationalOperator62/2
  Constant99/1 -> RelationalOperator62/1
  RelationalOperator62/1 -> LogicalOperator27/1
  Constant13/1 -> RelationalOperator63/2
  Constant101/1 -> RelationalOperator63/1
  RelationalOperator63/1 -> LogicalOperator30/1
  Constant90/1 -> RelationalOperator67/1
  Constant8/1 -> RelationalOperator67/2
  RelationalOperator67/1 -> LogicalOperator32/1
  Constant102/1 -> RelationalOperator64/1
  Constant15/1 -> RelationalOperator64/2
  RelationalOperator64/1 -> LogicalOperator34/1
  Logical Operator27/1 -> LogicalOperator27/2
  Logical Operator45/1 -> LogicalOperator48/2
  Constant121/1 -> RelationalOperator76/2
  RelationalOperator76/1 -> Logical Operator27/1
  Constant118/1 -> RelationalOperator75/2
  Constant119/1 -> RelationalOperator77/2
  RelationalOperator77/1 -> Logical Operator30/1
  Logical Operator30/1 -> LogicalOperator34/2
  Logical Operator29/1 -> LogicalOperator32/2
  Constant120/1 -> RelationalOperator80/2
  Logical Operator31/1 -> LogicalOperator30/2
  RelationalOperator82/1 -> Logical Operator45/1
  RelationalOperator75/1 -> Logical Operator29/1
  RelationalOperator80/1 -> Logical Operator31/1
  [Number_Subfunction] -> RelationalOperator82/1
  [Number_Subfunction] -> RelationalOperator76/1
  [Number_Subfunction] -> RelationalOperator80/1
  [Number_Subfunction] -> RelationalOperator75/1
  [Number_Subfunction] -> RelationalOperator77/1
  Constant123/1 -> RelationalOperator78/1
  Constant18/1 -> RelationalOperator78/2
  RelationalOperator78/1 -> LogicalOperator31/1
  Constant125/1 -> RelationalOperator79/2
  RelationalOperator79/1 -> Logical Operator28/1
  Logical Operator28/1 -> LogicalOperator31/2
  [Number_Subfunction] -> RelationalOperator79/1
  Constant117/1 -> RelationalOperator74/2
  Constant107/1 -> RelationalOperator83/1
  Constant19/1 -> RelationalOperator83/2
  RelationalOperator83/1 -> LogicalOperator33/1
  Constant20/1 -> RelationalOperator68/2
  Constant109/1 -> RelationalOperator68/1
  RelationalOperator68/1 -> LogicalOperator35/1
  Constant22/1 -> RelationalOperator69/2
  Constant111/1 -> RelationalOperator69/1
  RelationalOperator69/1 -> LogicalOperator36/1
  Constant127/1 -> RelationalOperator70/1
  Constant27/1 -> RelationalOperator70/2
  RelationalOperator70/1 -> LogicalOperator29/1
  Logical Operator33/1 -> LogicalOperator35/2
  Logical Operator32/1 -> LogicalOperator33/2
  Constant116/1 -> RelationalOperator72/2
  RelationalOperator72/1 -> Logical Operator33/1
  Constant113/1 -> RelationalOperator71/2
  Logical Operator34/1 -> LogicalOperator29/2
  Constant114/1 -> RelationalOperator73/2
  Logical Operator25/1 -> LogicalOperator36/2
  RelationalOperator74/1 -> Logical Operator32/1
  RelationalOperator71/1 -> Logical Operator34/1
  RelationalOperator73/1 -> Logical Operator25/1
  [Number_Subfunction] -> RelationalOperator74/1
  [Number_Subfunction] -> RelationalOperator72/1
  [Number_Subfunction] -> RelationalOperator73/1
  [Number_Subfunction] -> RelationalOperator71/1
  Constant28/1 -> RelationalOperator84/2
  Constant129/1 -> RelationalOperator84/1
  RelationalOperator84/1 -> LogicalOperator39/1
  Constant34/1 -> RelationalOperator85/2
  Constant131/1 -> RelationalOperator85/1
  RelationalOperator85/1 -> LogicalOperator40/1
  Constant138/1 -> RelationalOperator86/1
  Constant36/1 -> RelationalOperator86/2
  RelationalOperator86/1 -> LogicalOperator38/1
  Logical Operator37/1 -> LogicalOperator39/2
  Constant136/1 -> RelationalOperator89/2
  RelationalOperator89/1 -> Logical Operator37/1
  Constant134/1 -> RelationalOperator88/2
  Logical Operator38/1 -> LogicalOperator38/2
  Constant135/1 -> RelationalOperator90/2
  Logical Operator36/1 -> LogicalOperator40/2
  RelationalOperator88/1 -> Logical Operator38/1
  RelationalOperator90/1 -> Logical Operator36/1
  [Number_Subfunction] -> RelationalOperator89/1
  [Number_Subfunction] -> RelationalOperator90/1
  [Number_Subfunction] -> RelationalOperator88/1
  LogicalOperator23/1 -> LogicalOperator43/1
  LogicalOperator25/1 -> LogicalOperator43/2
  LogicalOperator37/1 -> LogicalOperator43/3
  LogicalOperator42/1 -> LogicalOperator43/4
  LogicalOperator45/1 -> LogicalOperator43/5
  LogicalOperator41/1 -> LogicalOperator43/6
  LogicalOperator44/1 -> LogicalOperator43/7
  LogicalOperator46/1 -> LogicalOperator43/8
  LogicalOperator47/1 -> LogicalOperator43/9
  LogicalOperator26/1 -> LogicalOperator43/10
  LogicalOperator48/1 -> LogicalOperator43/11
  LogicalOperator27/1 -> LogicalOperator43/12
  LogicalOperator30/1 -> LogicalOperator43/13
  LogicalOperator32/1 -> LogicalOperator43/14
  LogicalOperator34/1 -> LogicalOperator43/15
  LogicalOperator31/1 -> LogicalOperator43/16
  LogicalOperator33/1 -> LogicalOperator43/17
  LogicalOperator35/1 -> LogicalOperator43/18
  LogicalOperator36/1 -> LogicalOperator43/19
  LogicalOperator29/1 -> LogicalOperator43/20
  LogicalOperator39/1 -> LogicalOperator43/21
  LogicalOperator40/1 -> LogicalOperator43/22
  LogicalOperator38/1 -> LogicalOperator43/23
  LogicalOperator43/1 -> Out1/1
  Number_Subfunction/1 -> [Number_Subfunction]
